simulink model "dss_ideal_16384ch_32bits_reset_1966mhz_cx"  (R2021a) (continued)
== system dss_ideal_16384ch_32bits_reset_1966mhz_cx == (continued)
      mask init:
      | shared_bram_mask;
      mask TypeOptions arith_type: Unsigned | Signed  (2's comp)
      mask TypeOptions data_width: 8 | 16 | 32 | 64 | 128
      mask TypeOptions optimization: Minimum_Area | Low_Power
  synth0_2 : Subsystem  Tag=xps:bram  AncestorBlock=xps_library/Memory/shared_bram  mask{arith_type=Unsigned, addr_width=11, data_width=32, reg_prim_output=on, reg_core_output=on, optimization=Minimum_Area, data_bin_pt=0, init_vals=[0:2^11-1], sample_rate=1}
      mask prompts: arith_type "Output Data Type"; addr_width "Address width"; data_width "Data Width"; reg_prim_output "Register Primitive Output"; reg_core_output "Register Core Output"; data_bin_pt "Data Binary Point"; init_vals "Initial values (simulation only)"; sample_rate "Sample rate"
      mask init:
      | shared_bram_mask;
      mask TypeOptions arith_type: Unsigned | Signed  (2's comp)
      mask TypeOptions data_width: 8 | 16 | 32 | 64 | 128
      mask TypeOptions optimization: Minimum_Area | Low_Power
  synth0_3 : Subsystem  Tag=xps:bram  AncestorBlock=xps_library/Memory/shared_bram  mask{arith_type=Unsigned, addr_width=11, data_width=32, reg_prim_output=on, reg_core_output=on, optimization=Minimum_Area, data_bin_pt=0, init_vals=[0:2^11-1], sample_rate=1}
      mask prompts: arith_type "Output Data Type"; addr_width "Address width"; data_width "Data Width"; reg_prim_output "Register Primitive Output"; reg_core_output "Register Core Output"; data_bin_pt "Data Binary Point"; init_vals "Initial values (simulation only)"; sample_rate "Sample rate"
      mask init:
      | shared_bram_mask;
      mask TypeOptions arith_type: Unsigned | Signed  (2's comp)
      mask TypeOptions data_width: 8 | 16 | 32 | 64 | 128
      mask TypeOptions optimization: Minimum_Area | Low_Power
  synth0_4 : Subsystem  Tag=xps:bram  AncestorBlock=xps_library/Memory/shared_bram  mask{arith_type=Unsigned, addr_width=11, data_width=32, reg_prim_output=on, reg_core_output=on, optimization=Minimum_Area, data_bin_pt=0, init_vals=[0:2^11-1], sample_rate=1}
      mask prompts: arith_type "Output Data Type"; addr_width "Address width"; data_width "Data Width"; reg_prim_output "Register Primitive Output"; reg_core_output "Register Core Output"; data_bin_pt "Data Binary Point"; init_vals "Initial values (simulation only)"; sample_rate "Sample rate"
      mask init:
      | shared_bram_mask;
      mask TypeOptions arith_type: Unsigned | Signed  (2's comp)
      mask TypeOptions data_width: 8 | 16 | 32 | 64 | 128
      mask TypeOptions optimization: Minimum_Area | Low_Power
  synth0_5 : Subsystem  Tag=xps:bram  AncestorBlock=xps_library/Memory/shared_bram  mask{arith_type=Unsigned, addr_width=11, data_width=32, reg_prim_output=on, reg_core_output=on, optimization=Minimum_Area, data_bin_pt=0, init_vals=[0:2^11-1], sample_rate=1}
      mask prompts: arith_type "Output Data Type"; addr_width "Address width"; data_width "Data Width"; reg_prim_output "Register Primitive Output"; reg_core_output "Register Core Output"; data_bin_pt "Data Binary Point"; init_vals "Initial values (simulation only)"; sample_rate "Sample rate"
      mask init:
      | shared_bram_mask;
      mask TypeOptions arith_type: Unsigned | Signed  (2's comp)
      mask TypeOptions data_width: 8 | 16 | 32 | 64 | 128
      mask TypeOptions optimization: Minimum_Area | Low_Power
  synth0_6 : Subsystem  Tag=xps:bram  AncestorBlock=xps_library/Memory/shared_bram  mask{arith_type=Unsigned, addr_width=11, data_width=32, reg_prim_output=on, reg_core_output=on, optimization=Minimum_Area, data_bin_pt=0, init_vals=[0:2^11-1], sample_rate=1}
      mask prompts: arith_type "Output Data Type"; addr_width "Address width"; data_width "Data Width"; reg_prim_output "Register Primitive Output"; reg_core_output "Register Core Output"; data_bin_pt "Data Binary Point"; init_vals "Initial values (simulation only)"; sample_rate "Sample rate"
      mask init:
      | shared_bram_mask;
      mask TypeOptions arith_type: Unsigned | Signed  (2's comp)
      mask TypeOptions data_width: 8 | 16 | 32 | 64 | 128
      mask TypeOptions optimization: Minimum_Area | Low_Power
  synth0_7 : Subsystem  Tag=xps:bram  AncestorBlock=xps_library/Memory/shared_bram  mask{arith_type=Unsigned, addr_width=11, data_width=32, reg_prim_output=on, reg_core_output=on, optimization=Minimum_Area, data_bin_pt=0, init_vals=[0:2^11-1], sample_rate=1}
      mask prompts: arith_type "Output Data Type"; addr_width "Address width"; data_width "Data Width"; reg_prim_output "Register Primitive Output"; reg_core_output "Register Core Output"; data_bin_pt "Data Binary Point"; init_vals "Initial values (simulation only)"; sample_rate "Sample rate"
      mask init:
      | shared_bram_mask;
      mask TypeOptions arith_type: Unsigned | Signed  (2's comp)
      mask TypeOptions data_width: 8 | 16 | 32 | 64 | 128
      mask TypeOptions optimization: Minimum_Area | Low_Power
  synth1_0 : Subsystem  Tag=xps:bram  AncestorBlock=xps_library/Memory/shared_bram  mask{arith_type=Unsigned, addr_width=11, data_width=32, reg_prim_output=on, reg_core_output=on, optimization=Minimum_Area, data_bin_pt=0, init_vals=[0:2^11-1], sample_rate=1}
      mask prompts: arith_type "Output Data Type"; addr_width "Address width"; data_width "Data Width"; reg_prim_output "Register Primitive Output"; reg_core_output "Register Core Output"; data_bin_pt "Data Binary Point"; init_vals "Initial values (simulation only)"; sample_rate "Sample rate"
      mask init:
      | shared_bram_mask;
      mask TypeOptions arith_type: Unsigned | Signed  (2's comp)
      mask TypeOptions data_width: 8 | 16 | 32 | 64 | 128
      mask TypeOptions optimization: Minimum_Area | Low_Power
  synth1_1 : Subsystem  Tag=xps:bram  AncestorBlock=xps_library/Memory/shared_bram  mask{arith_type=Unsigned, addr_width=11, data_width=32, reg_prim_output=on, reg_core_output=on, optimization=Minimum_Area, data_bin_pt=0, init_vals=[0:2^11-1], sample_rate=1}
      mask prompts: arith_type "Output Data Type"; addr_width "Address width"; data_width "Data Width"; reg_prim_output "Register Primitive Output"; reg_core_output "Register Core Output"; data_bin_pt "Data Binary Point"; init_vals "Initial values (simulation only)"; sample_rate "Sample rate"
      mask init:
      | shared_bram_mask;
      mask TypeOptions arith_type: Unsigned | Signed  (2's comp)
      mask TypeOptions data_width: 8 | 16 | 32 | 64 | 128
      mask TypeOptions optimization: Minimum_Area | Low_Power
  synth1_2 : Subsystem  Tag=xps:bram  AncestorBlock=xps_library/Memory/shared_bram  mask{arith_type=Unsigned, addr_width=11, data_width=32, reg_prim_output=on, reg_core_output=on, optimization=Minimum_Area, data_bin_pt=0, init_vals=[0:2^11-1], sample_rate=1}
      mask prompts: arith_type "Output Data Type"; addr_width "Address width"; data_width "Data Width"; reg_prim_output "Register Primitive Output"; reg_core_output "Register Core Output"; data_bin_pt "Data Binary Point"; init_vals "Initial values (simulation only)"; sample_rate "Sample rate"
      mask init:
      | shared_bram_mask;
      mask TypeOptions arith_type: Unsigned | Signed  (2's comp)
      mask TypeOptions data_width: 8 | 16 | 32 | 64 | 128
      mask TypeOptions optimization: Minimum_Area | Low_Power
  synth1_3 : Subsystem  Tag=xps:bram  AncestorBlock=xps_library/Memory/shared_bram  mask{arith_type=Unsigned, addr_width=11, data_width=32, reg_prim_output=on, reg_core_output=on, optimization=Minimum_Area, data_bin_pt=0, init_vals=[0:2^11-1], sample_rate=1}
      mask prompts: arith_type "Output Data Type"; addr_width "Address width"; data_width "Data Width"; reg_prim_output "Register Primitive Output"; reg_core_output "Register Core Output"; data_bin_pt "Data Binary Point"; init_vals "Initial values (simulation only)"; sample_rate "Sample rate"
      mask init:
      | shared_bram_mask;
      mask TypeOptions arith_type: Unsigned | Signed  (2's comp)
      mask TypeOptions data_width: 8 | 16 | 32 | 64 | 128
      mask TypeOptions optimization: Minimum_Area | Low_Power
  synth1_4 : Subsystem  Tag=xps:bram  AncestorBlock=xps_library/Memory/shared_bram  mask{arith_type=Unsigned, addr_width=11, data_width=32, reg_prim_output=on, reg_core_output=on, optimization=Minimum_Area, data_bin_pt=0, init_vals=[0:2^11-1], sample_rate=1}
      mask prompts: arith_type "Output Data Type"; addr_width "Address width"; data_width "Data Width"; reg_prim_output "Register Primitive Output"; reg_core_output "Register Core Output"; data_bin_pt "Data Binary Point"; init_vals "Initial values (simulation only)"; sample_rate "Sample rate"
      mask init:
      | shared_bram_mask;
      mask TypeOptions arith_type: Unsigned | Signed  (2's comp)
      mask TypeOptions data_width: 8 | 16 | 32 | 64 | 128
      mask TypeOptions optimization: Minimum_Area | Low_Power
  synth1_5 : Subsystem  Tag=xps:bram  AncestorBlock=xps_library/Memory/shared_bram  mask{arith_type=Unsigned, addr_width=11, data_width=32, reg_prim_output=on, reg_core_output=on, optimization=Minimum_Area, data_bin_pt=0, init_vals=[0:2^11-1], sample_rate=1}
      mask prompts: arith_type "Output Data Type"; addr_width "Address width"; data_width "Data Width"; reg_prim_output "Register Primitive Output"; reg_core_output "Register Core Output"; data_bin_pt "Data Binary Point"; init_vals "Initial values (simulation only)"; sample_rate "Sample rate"
      mask init:
      | shared_bram_mask;
      mask TypeOptions arith_type: Unsigned | Signed  (2's comp)
      mask TypeOptions data_width: 8 | 16 | 32 | 64 | 128
      mask TypeOptions optimization: Minimum_Area | Low_Power
  synth1_6 : Subsystem  Tag=xps:bram  AncestorBlock=xps_library/Memory/shared_bram  mask{arith_type=Unsigned, addr_width=11, data_width=32, reg_prim_output=on, reg_core_output=on, optimization=Minimum_Area, data_bin_pt=0, init_vals=[0:2^11-1], sample_rate=1}
      mask prompts: arith_type "Output Data Type"; addr_width "Address width"; data_width "Data Width"; reg_prim_output "Register Primitive Output"; reg_core_output "Register Core Output"; data_bin_pt "Data Binary Point"; init_vals "Initial values (simulation only)"; sample_rate "Sample rate"
      mask init:
      | shared_bram_mask;
      mask TypeOptions arith_type: Unsigned | Signed  (2's comp)
      mask TypeOptions data_width: 8 | 16 | 32 | 64 | 128
      mask TypeOptions optimization: Minimum_Area | Low_Power
  synth1_7 : Subsystem  Tag=xps:bram  AncestorBlock=xps_library/Memory/shared_bram  mask{arith_type=Unsigned, addr_width=11, data_width=32, reg_prim_output=on, reg_core_output=on, optimization=Minimum_Area, data_bin_pt=0, init_vals=[0:2^11-1], sample_rate=1}
      mask prompts: arith_type "Output Data Type"; addr_width "Address width"; data_width "Data Width"; reg_prim_output "Register Primitive Output"; reg_core_output "Register Core Output"; data_bin_pt "Data Binary Point"; init_vals "Initial values (simulation only)"; sample_rate "Sample rate"
      mask init:
      | shared_bram_mask;
      mask TypeOptions arith_type: Unsigned | Signed  (2's comp)
      mask TypeOptions data_width: 8 | 16 | 32 | 64 | 128
      mask TypeOptions optimization: Minimum_Area | Low_Power
  wires:
  rfdc/1(m00_axis_tdata) -> bus_create/1(in1)
  rfdc/2(m01_axis_tdata) -> bus_create/2(in2)
  bus_create/1(bus_out) -> munge1/1(din), bus_expand2/1(bus_in)
  bus_expand/8(lsb_out1) -> pfb_fir/9(pol1_in8)
  bus_expand/1(msb_out8) -> pfb_fir/2(pol1_in1)
  bus_expand/3(out6) -> pfb_fir/4(pol1_in3)
  pfb_fir/7(pol1_out6) -> fft/8(in05)
  pfb_fir/1(sync_out) -> fft/1(sync)
  pfb_fir/9(pol1_out8) -> fft/10(in07)
  bus_expand/4(out5) -> pfb_fir/5(pol1_in4)
  [sync_gen] -> pfb_fir/1(sync)
  pfb_fir/5(pol1_out4) -> fft/6(in03)
  Constant2/1 -> fft/2(shift)
  bus_expand/7(out2) -> pfb_fir/8(pol1_in7)
  bus_expand/5(out4) -> pfb_fir/6(pol1_in5)
  bus_expand/6(out3) -> pfb_fir/7(pol1_in6)
  pfb_fir/6(pol1_out5) -> fft/7(in04)
  bus_expand/2(out7) -> pfb_fir/3(pol1_in2)
  pfb_fir/4(pol1_out3) -> fft/5(in02)
  pfb_fir/2(pol1_out1) -> fft/3(in00)
  pfb_fir/8(pol1_out7) -> fft/9(in06)
  pfb_fir/3(pol1_out2) -> fft/4(in01)
  Band-Limited White Noise/1 -> rfdc/10(m23_axis_tdata_sim), rfdc/11(m30_axis_tdata_sim), rfdc/12(m32_axis_tdata_sim), rfdc/9(m22_axis_tdata_sim), rfdc/8(m21_axis_tdata_sim), rfdc/7(m20_axis_tdata_sim), rfdc/6(m12_axis_tdata_sim), rfdc/5(m10_axis_tdata_sim), rfdc/4(m03_axis_tdata_sim), rfdc/3(m02_axis_tdata_sim), rfdc/2(m01_axis_tdata_sim), rfdc/1(m00_axis_tdata_sim)
  rfdc/5(m10_axis_tdata) -> Terminator4/1
  rfdc/6(m12_axis_tdata) -> Terminator9/1
  rfdc/8(m21_axis_tdata) -> Terminator17/1
  rfdc/12(m32_axis_tdata) -> Terminator19/1
  rfdc/7(m20_axis_tdata) -> Terminator16/1
  rfdc/9(m22_axis_tdata) -> Terminator20/1
  rfdc/10(m23_axis_tdata) -> Terminator21/1
  rfdc/11(m30_axis_tdata) -> Terminator18/1
  pipeline157/1(q) -> comp_add/1(a)
  pipeline113/1(q) -> spect_cnt2/1
  simple_bram_vacc1/2(valid) -> spect_cnt2/2, pipeline118/1(d)
  pipeline161/1(q) -> Delay46/1
  pipeline126/1(q) -> simple_bram_vacc1/2(din)
  bram_mult0_0/1(c) -> comp_add/2(b)
  Delay46/1 -> Delay43/1
  Reinterpret21/1 -> pipeline117/1(d)
  simple_bram_vacc1/1(dout) -> Reinterpret21/1
  pipeline118/1(q) -> synth0_0/3(we)
  pipeline162/1(q) -> pipeline161/1(d)
  synth0_0/1(data_out) -> Terminator1/1
  pipeline125/1(q) -> simple_bram_vacc1/1(new_acc)
  comp_add/1(a+b) -> power1/1
  pipeline104/1(q) -> synth0_0/1(addr)
  [acc_len] -> acc_cntrl/1(acc_len)
  [sync_rst] -> acc_cntrl/3(rst)
  pipeline117/1(q) -> synth0_0/2(data_in)
  power1/1 -> Delay44/1
  fft/1(sync_out) -> Delay39/1
  Delay39/1 -> Delay38/1
  Delay38/1 -> pipeline162/1(d), bram_mult0_1/1(In1), bram_mult0_2/1(In1), bram_mult0_3/1(In1), bram_mult0_4/1(In1), bram_mult0_5/1(In1), bram_mult0_8/1(In1), bram_mult0_9/1(In1), bram_mult0_10/1(In1), bram_mult0_11/1(In1), bram_mult0_12/1(In1), bram_mult0_13/1(In1), bram_mult0_14/1(In1), bram_mult0_15/1(In1), bram_mult0_7/1(In1), bram_mult0_6/1(In1), bram_mult0_0/1(In1)
  Delay15/1 -> bram_mult0_8/2(d), pipeline157/1(d)
  fft/2(out00) -> Delay18/1
  Delay18/1 -> Delay15/1
  Delay3/1 -> bram_mult0_9/2(d), pipeline10/1(d)
  fft/3(out01) -> Delay4/1
  Delay4/1 -> Delay3/1
  Delay2/1 -> Delay1/1
  fft/4(out02) -> Delay2/1
  Delay1/1 -> bram_mult0_10/2(d), pipeline12/1(d)
  fft/6(out04) -> Delay6/1
  Delay8/1 -> Delay7/1
  Delay6/1 -> Delay5/1
  fft/5(out03) -> Delay8/1
  Delay5/1 -> bram_mult0_12/2(d), pipeline21/1(d)
  Delay7/1 -> bram_mult0_11/2(d), pipeline19/1(d)
  Delay10/1 -> Delay9/1
  Delay12/1 -> Delay11/1
  Delay13/1 -> bram_mult0_14/2(d), pipeline40/1(d)
  Delay11/1 -> bram_mult0_15/2(d), pipeline42/1(d)
  Delay9/1 -> bram_mult0_13/2(d), pipeline33/1(d)
  Delay14/1 -> Delay13/1
  pipeline1/1(q) -> synth0_1/1(addr)
  pipeline4/1(q) -> synth0_1/3(we)
  simple_bram_vacc2/1(dout) -> Reinterpret1/1
  pipeline2/1(q) -> synth0_1/2(data_in)
  pipeline6/1(q) -> simple_bram_vacc2/1(new_acc)
  bram_mult0_1/1(c) -> comp_add1/2(b)
  pipeline10/1(q) -> comp_add1/1(a)
  power2/1 -> Delay16/1
  synth0_1/1(data_out) -> Terminator3/1
  simple_bram_vacc2/2(valid) -> pipeline4/1(d)
  comp_add1/1(a+b) -> power2/1
  pipeline7/1(q) -> simple_bram_vacc2/2(din)
  Reinterpret1/1 -> pipeline2/1(d)
  Reinterpret2/1 -> pipeline13/1(d)
  pipeline12/1(q) -> comp_add2/1(a)
  simple_bram_vacc3/2(valid) -> pipeline14/1(d)
  power3/1 -> Delay17/1
  bram_mult0_2/1(c) -> comp_add2/2(b)
  synth0_2/1(data_out) -> Terminator5/1
  comp_add2/1(a+b) -> power3/1
  pipeline17/1(q) -> simple_bram_vacc3/2(din)
  pipeline13/1(q) -> synth0_2/2(data_in)
  simple_bram_vacc3/1(dout) -> Reinterpret2/1
  pipeline16/1(q) -> simple_bram_vacc3/1(new_acc)
  pipeline14/1(q) -> synth0_2/3(we)
  bram_mult0_4/1(c) -> comp_add4/2(b)
  pipeline27/1(q) -> synth0_3/2(data_in)
  comp_add4/1(a+b) -> power5/1
  pipeline31/1(q) -> simple_bram_vacc4/2(din)
  pipeline23/1(q) -> synth0_4/3(we)
  bram_mult0_3/1(c) -> comp_add3/2(b)
  Reinterpret3/1 -> pipeline27/1(d)
  pipeline19/1(q) -> comp_add3/1(a)
  power5/1 -> Delay20/1
  Reinterpret4/1 -> pipeline22/1(d)
  pipeline22/1(q) -> synth0_4/2(data_in)
  pipeline25/1(q) -> simple_bram_vacc5/1(new_acc)
  simple_bram_vacc4/2(valid) -> pipeline28/1(d)
  simple_bram_vacc5/2(valid) -> pipeline23/1(d)
  synth0_3/1(data_out) -> Terminator6/1
  synth0_4/1(data_out) -> Terminator7/1
  simple_bram_vacc4/1(dout) -> Reinterpret3/1
  pipeline30/1(q) -> simple_bram_vacc4/1(new_acc)
  power4/1 -> Delay19/1
  comp_add3/1(a+b) -> power4/1
  pipeline21/1(q) -> comp_add4/1(a)
  simple_bram_vacc5/1(dout) -> Reinterpret4/1
  pipeline26/1(q) -> simple_bram_vacc5/2(din)
  pipeline28/1(q) -> synth0_3/3(we)
  pipeline47/1(q) -> simple_bram_vacc8/2(din)
  pipeline51/1(q) -> simple_bram_vacc7/1(new_acc)
  simple_bram_vacc6/2(valid) -> pipeline35/1(d)
  pipeline33/1(q) -> comp_add5/1(a)
  simple_bram_vacc6/1(dout) -> Reinterpret5/1
  pipeline43/1(q) -> synth0_7/2(data_in)
  synth0_6/1(data_out) -> Terminator10/1
  power8/1 -> Delay24/1
  power7/1 -> Delay22/1
  simple_bram_vacc8/2(valid) -> pipeline44/1(d)
  simple_bram_vacc8/1(dout) -> Reinterpret7/1
  Reinterpret5/1 -> pipeline34/1(d)
  bram_mult0_5/1(c) -> comp_add5/2(b)
  comp_add6/1(a+b) -> power7/1
  pipeline48/1(q) -> synth0_6/2(data_in)
  simple_bram_vacc7/1(dout) -> Reinterpret6/1
  simple_bram_vacc7/2(valid) -> pipeline49/1(d)
  bram_mult0_7/1(c) -> comp_add7/2(b)
  pipeline44/1(q) -> synth0_7/3(we)
  pipeline34/1(q) -> synth0_5/2(data_in)
  synth0_7/1(data_out) -> Terminator11/1
  pipeline42/1(q) -> comp_add7/1(a)
  bram_mult0_6/1(c) -> comp_add6/2(b)
  pipeline52/1(q) -> simple_bram_vacc7/2(din)
  Reinterpret6/1 -> pipeline48/1(d)
  synth0_5/1(data_out) -> Terminator8/1
  pipeline37/1(q) -> simple_bram_vacc6/1(new_acc)
  pipeline46/1(q) -> simple_bram_vacc8/1(new_acc)
  Reinterpret7/1 -> pipeline43/1(d)
  pipeline35/1(q) -> synth0_5/3(we)
  comp_add5/1(a+b) -> power6/1
  pipeline49/1(q) -> synth0_6/3(we)
  comp_add7/1(a+b) -> power8/1
  pipeline38/1(q) -> simple_bram_vacc6/2(din)
  pipeline40/1(q) -> comp_add6/1(a)
  power6/1 -> Delay21/1
  Delay57/1 -> Delay56/1
  simple_bram_vacc13/1(dout) -> Reinterpret12/1
  pipeline76/1(q) -> comp_add12/1(a)
  fft/15(out15) -> Delay26/1
  Delay55/1 -> Delay54/1
  pipeline99/1(q) -> comp_add15/1(a)
  bus_expand1/2(out7) -> pfb_fir/11(pol2_in2)
  synth1_5/1(data_out) -> Terminator25/1
  bram_mult0_14/1(c) -> comp_add14/2(b)
  power13/1 -> Delay37/1
  simple_bram_vacc16/1(dout) -> Reinterpret15/1
  fft/16(out16) -> Delay30/1
  pipeline94/1(q) -> simple_bram_vacc14/2(din)
  fft/11(out11) -> Delay50/1
  Delay35/1 -> Delay220/1
  pipeline55/1(q) -> synth1_0/1(addr)
  pipeline80/1(q) -> simple_bram_vacc13/1(new_acc)
  pipeline89/1(q) -> comp_add13/1(a)
  Delay58/1 -> bram_mult0_5/2(d), pipeline89/1(d)
  pipeline86/1(q) -> simple_bram_vacc12/1(new_acc)
  Delay54/1 -> bram_mult0_4/2(d), pipeline76/1(d)
  pipeline97/1(q) -> comp_add14/1(a)
  simple_bram_vacc9/1(dout) -> Reinterpret10/1
  simple_bram_vacc10/1(dout) -> Reinterpret8/1
  pfb_fir/11(pol2_out2) -> fft/12(in11)
  Delay34/1 -> Delay31/1
  power14/1 -> Delay40/1
  simple_bram_vacc14/2(valid) -> pipeline91/1(d)
  simple_bram_vacc15/1(dout) -> Reinterpret14/1
  simple_bram_vacc9/2(valid) -> pipeline59/1(d)
  Delay41/1 -> Delay222/1
  simple_bram_vacc12/2(valid) -> pipeline83/1(d)
  power15/1 -> Delay41/1
  Reinterpret13/1 -> pipeline90/1(d)
  Delay33/1 -> Delay219/1
  fft/10(out10) -> Delay34/1
  bus_expand1/8(lsb_out1) -> pfb_fir/17(pol2_in8)
  simple_bram_vacc13/2(valid) -> pipeline78/1(d)
  pipeline62/1(q) -> simple_bram_vacc9/1(new_acc)
  bram_mult0_11/1(c) -> comp_add11/2(b)
  Reinterpret9/1 -> pipeline64/1(d)
  Delay37/1 -> Delay221/1
  pipeline105/1(q) -> simple_bram_vacc16/2(din)
  pipeline87/1(q) -> simple_bram_vacc12/2(din)
  simple_bram_vacc16/2(valid) -> pipeline101/1(d)
  pfb_fir/12(pol2_out3) -> fft/13(in12)
  pipeline68/1(q) -> simple_bram_vacc11/1(new_acc)
  power12/1 -> Delay35/1
  pfb_fir/17(pol2_out8) -> fft/18(in17)
  fft/12(out12) -> Delay36/1
  pipeline65/1(q) -> synth1_2/3(we)
  pipeline91/1(q) -> synth1_5/3(we)
  Reinterpret8/1 -> pipeline74/1(d)
  bram_mult0_12/1(c) -> comp_add12/2(b)
  bus_expand1/3(out6) -> pfb_fir/12(pol2_in3)
  fft/14(out14) -> Delay55/1
  pipeline90/1(q) -> synth1_5/2(data_in)
  pipeline107/1(q) -> synth1_6/3(we)
  fft/17(out17) -> Delay28/1
  synth1_1/1(data_out) -> Terminator15/1
  pfb_fir/14(pol2_out5) -> fft/15(in14)
  bram_mult0_10/1(c) -> comp_add10/2(b)
  pipeline106/1(q) -> synth1_6/2(data_in)
  pipeline63/1(q) -> simple_bram_vacc9/2(din)
  Reinterpret14/1 -> pipeline106/1(d)
  pipeline71/1(q) -> simple_bram_vacc11/2(din)
  simple_bram_vacc11/2(valid) -> pipeline65/1(d)
  Delay56/1 -> bram_mult0_3/2(d), pipeline73/1(d)
  pipeline59/1(q) -> synth1_0/3(we)
  pipeline78/1(q) -> synth1_4/3(we)
  bram_mult0_8/1(c) -> comp_add8/2(b)
  simple_bram_vacc15/2(valid) -> pipeline107/1(d)
  pfb_fir/13(pol2_out4) -> fft/14(in13)
  bram_mult0_13/1(c) -> comp_add13/2(b)
  pipeline77/1(q) -> synth1_4/2(data_in)
  bus_expand1/5(out4) -> pfb_fir/14(pol2_in5)
  bus_expand1/1(msb_out8) -> pfb_fir/10(pol2_in1)
  Delay29/1 -> bram_mult0_6/2(d), pipeline97/1(d)
  Delay27/1 -> pipeline99/1(d), bram_mult0_7/2(d)
  Delay50/1 -> Delay47/1
  Reinterpret11/1 -> pipeline82/1(d)
  synth1_7/1(data_out) -> Terminator14/1
  Delay26/1 -> Delay58/1
  Reinterpret15/1 -> pipeline100/1(d)
  pipeline82/1(q) -> synth1_3/2(data_in)
  power9/1 -> Delay52/1
  simple_bram_vacc14/1(dout) -> Reinterpret13/1
  pipeline54/1(q) -> comp_add9/1(a)
  Reinterpret10/1 -> pipeline58/1(d)
  pipeline64/1(q) -> synth1_2/2(data_in)
  power10/1 -> Delay32/1
  Delay47/1 -> bram_mult0_1/2(d), pipeline54/1(d)
  pipeline101/1(q) -> synth1_7/3(we)
  bram_mult0_15/1(c) -> comp_add15/2(b)
  simple_bram_vacc12/1(dout) -> Reinterpret11/1
  power11/1 -> Delay33/1
  fft/13(out13) -> Delay57/1
  Delay36/1 -> Delay25/1
  Reinterpret12/1 -> pipeline77/1(d)
  synth1_2/1(data_out) -> Terminator22/1
  pipeline93/1(q) -> simple_bram_vacc14/1(new_acc)
  Delay30/1 -> Delay29/1
  simple_bram_vacc10/2(valid) -> pipeline96/1(d)
  Delay45/1 -> Delay225/1
  pipeline67/1(q) -> comp_add8/1(a)
  Delay40/1 -> Delay226/1
  bus_expand1/7(out2) -> pfb_fir/16(pol2_in7)
  synth1_6/1(data_out) -> Terminator13/1
  pipeline58/1(q) -> synth1_0/2(data_in)
  pipeline100/1(q) -> synth1_7/2(data_in)
  synth1_3/1(data_out) -> Terminator23/1
  pipeline53/1(q) -> synth1_1/1(addr)
  pipeline60/1(q) -> comp_add10/1(a)
  power16/1 -> Delay45/1
  pipeline114/1(q) -> simple_bram_vacc10/2(din)
  bus_expand1/6(out3) -> pfb_fir/15(pol2_in6)
  Delay31/1 -> bram_mult0_0/2(d), pipeline67/1(d)
  pipeline81/1(q) -> simple_bram_vacc13/2(din)
  bram_mult0_9/1(c) -> comp_add9/2(b)
  simple_bram_vacc11/1(dout) -> Reinterpret9/1
  pipeline83/1(q) -> synth1_3/3(we)
  pipeline112/1(q) -> simple_bram_vacc10/1(new_acc)
  synth1_4/1(data_out) -> Terminator24/1
  Delay28/1 -> Delay27/1
  pipeline103/1(q) -> simple_bram_vacc16/1(new_acc)
  Delay25/1 -> bram_mult0_2/2(d), pipeline60/1(d)
  pfb_fir/10(pol2_out1) -> fft/11(in10)
  pipeline74/1(q) -> synth1_1/2(data_in)
  pipeline73/1(q) -> comp_add11/1(a)
  pfb_fir/16(pol2_out7) -> fft/17(in16)
  pipeline111/1(q) -> simple_bram_vacc15/2(din)
  pfb_fir/15(pol2_out6) -> fft/16(in15)
  pipeline110/1(q) -> simple_bram_vacc15/1(new_acc)
  pipeline96/1(q) -> synth1_1/3(we)
  bus_expand1/4(out5) -> pfb_fir/13(pol2_in4)
  synth1_0/1(data_out) -> Terminator12/1
  rfdc/3(m02_axis_tdata) -> bus_create1/1(in1)
  rfdc/4(m03_axis_tdata) -> bus_create1/2(in2)
  pipeline11/1(q) -> synth0_2/1(addr)
  pipeline18/1(q) -> synth0_3/1(addr)
  pipeline20/1(q) -> synth0_4/1(addr)
  pipeline32/1(q) -> synth0_5/1(addr)
  pipeline39/1(q) -> synth0_6/1(addr)
  pipeline41/1(q) -> synth0_7/1(addr)
  pipeline56/1(q) -> synth1_2/1(addr)
  pipeline72/1(q) -> synth1_3/1(addr)
  pipeline75/1(q) -> synth1_4/1(addr)
  pipeline88/1(q) -> synth1_5/1(addr)
  pipeline95/1(q) -> synth1_6/1(addr)
  pipeline98/1(q) -> synth1_7/1(addr)
  adc1_snapshot0/1(arm_out) -> Terminator28/1
  Reinterpret17/1 -> adc1_snapshot0/1(in_m00)
  bus_expand2/2(lsb_out1) -> Reinterpret16/1
  adc1_snapshot1/1(arm_out) -> Terminator29/1
  bus_expand2/1(msb_out2) -> Reinterpret17/1
  Constant4/1 -> adc1_snapshot1/3(trig), adc1_snapshot1/2(we)
  Constant3/1 -> adc1_snapshot0/3(trig), adc1_snapshot0/2(we)
  Reinterpret18/1 -> adc1_snapshot3/1(in_m03)
  bus_expand3/2(lsb_out1) -> Reinterpret18/1
  adc1_snapshot2/1(arm_out) -> Terminator30/1
  bus_create1/1(bus_out) -> bus_expand3/1(bus_in), munge2/1(din)
  bus_expand3/1(msb_out2) -> Reinterpret19/1
  Reinterpret19/1 -> adc1_snapshot2/1(in_m02)
  Constant5/1 -> adc1_snapshot2/3(trig), adc1_snapshot2/2(we)
  Constant6/1 -> adc1_snapshot3/3(trig), adc1_snapshot3/2(we)
  adc1_snapshot3/1(arm_out) -> Terminator31/1
  comp_add9/1(a+b) -> power10/1
  comp_add8/1(a+b) -> power9/1
  comp_add10/1(a+b) -> power11/1
  comp_add11/1(a+b) -> power12/1
  comp_add12/1(a+b) -> power13/1
  comp_add13/1(a+b) -> power14/1
  comp_add14/1(a+b) -> power15/1
  comp_add15/1(a+b) -> power16/1
  Constant16/1 -> acc_len/1(sim_1)
  pipeline435/1(q) -> [cnt_rst]
  Constant19/1 -> cnt_rst/1(sim_1)
  cnt_rst/1(in_reg) -> pipeline435/1(d)
  acc_len/1(in_reg) -> pipeline9/1(d)
  pipeline9/1(q) -> [acc_len]
  pipeline121/1(q) -> pipeline126/1(d)
  pipeline5/1(q) -> pipeline7/1(d)
  pipeline15/1(q) -> pipeline17/1(d)
  pipeline29/1(q) -> pipeline31/1(d)
  pipeline24/1(q) -> pipeline26/1(d)
  pipeline45/1(q) -> pipeline47/1(d)
  pipeline36/1(q) -> pipeline38/1(d)
  pipeline50/1(q) -> pipeline52/1(d)
  pipeline108/1(q) -> pipeline114/1(d)
  pipeline102/1(q) -> pipeline105/1(d)
  pipeline109/1(q) -> pipeline111/1(d)
  pipeline79/1(q) -> pipeline81/1(d)
  pipeline92/1(q) -> pipeline94/1(d)
  pipeline66/1(q) -> pipeline71/1(d)
  pipeline84/1(q) -> pipeline87/1(d)
  pipeline61/1(q) -> pipeline63/1(d)
  Delay17/1 -> Delay155/1
  Delay154/1 -> round0/1(din)
  Delay53/1 -> round1/1(din)
  Delay20/1 -> Delay164/1
  Delay51/1 -> Delay223/1
  Delay19/1 -> Delay162/1
  Delay44/1 -> Delay154/1
  Delay24/1 -> Delay216/1
  Delay16/1 -> Delay53/1
  Delay155/1 -> round2/1(din)
  Delay224/1 -> Delay51/1
  Delay162/1 -> round3/1(din)
  Delay216/1 -> round7/1(din)
  Delay164/1 -> round4/1(din)
  Delay217/1 -> round5/1(din)
  [quant_gain] -> round0/2(scale), round1/2(scale), round2/2(scale), round3/2(scale), round4/2(scale), round5/2(scale), round6/2(scale), round7/2(scale), round8/2(scale), round9/2(scale), round10/2(scale), round11/2(scale), round12/2(scale), round13/2(scale), round14/2(scale), round15/2(scale)
  Delay223/1 -> acc_cntrl/2(sync)
  Delay21/1 -> Delay217/1
  Delay43/1 -> Delay224/1
  Delay22/1 -> Delay215/1
  Delay215/1 -> round6/1(din)
  round7/1(dout) -> pipeline45/1(d)
  round6/1(dout) -> pipeline50/1(d)
  round5/1(dout) -> pipeline36/1(d)
  round4/1(dout) -> pipeline24/1(d)
  round3/1(dout) -> pipeline29/1(d)
  round2/1(dout) -> pipeline15/1(d)
  round1/1(dout) -> pipeline5/1(d)
  round0/1(dout) -> pipeline121/1(d)
  gain/1(in_reg) -> pipeline57/1(d)
  Constant45/1 -> gain/1(sim_1)
  pipeline57/1(q) -> [quant_gain]
  round8/1(dout) -> pipeline61/1(d)
  Delay52/1 -> Delay218/1
  Delay32/1 -> Delay227/1
  round9/1(dout) -> pipeline108/1(d)
  round10/1(dout) -> pipeline66/1(d)
  round11/1(dout) -> pipeline84/1(d)
  round12/1(dout) -> pipeline79/1(d)
  round13/1(dout) -> pipeline92/1(d)
  round14/1(dout) -> pipeline109/1(d)
  round15/1(dout) -> pipeline102/1(d)
  Delay221/1 -> round12/1(din)
  Delay220/1 -> round11/1(din)
  Delay226/1 -> round13/1(din)
  Delay222/1 -> round14/1(din)
  Delay227/1 -> round9/1(din)
  Delay218/1 -> round8/1(din)
  Delay225/1 -> round15/1(din)
  Delay219/1 -> round10/1(din)
  Delay23/1 -> pipeline3/1(d)
  pipeline3/1(q) -> [sync_gen]
  sync_gen/1(sync_out) -> Delay23/1
  edge_detect/1(out) -> [sync_rst]
  Constant10/1 -> gpio/1
  gpio/1 -> edge_detect/1(in)
  [sync_rst] -> sync_cntr/2
  rst_cnt/1(sim_out_reg) -> Terminator85/1
  sync_cntr/1 -> Delay107/1
  Delay107/1 -> rst_cnt/1(out_reg)
  pipeline/1(q) -> pulse_ext1/1(in)
  [cnt_rst] -> sync_cntr/1
  pulse_ext1/1(out) -> pipeline219/1(d)
  [sync_rst] -> pipeline/1(d)
  led0_sync/1 -> Terminator84/1
  pipeline219/1(q) -> led0_sync/1
  [sync_rst] -> acc_cycle/1
  acc_cnt/1(sim_out_reg) -> Terminator86/1
  acc_cntr/1 -> Delay133/1
  Delay213/1 -> Relational1/1, Register2/1, acc_per_cycle/1(out_reg)
  Constant13/1 -> Relational4/1
  Register2/1 -> Relational4/2
  Relational1/1 -> Logical/2
  [cnt_rst] -> acc_cntr/1
  Logical/1 -> acc_cntr/2
  acc_per_cycle/1(sim_out_reg) -> Terminator81/1
  acc_cycle/1 -> Delay213/1
  Delay214/1 -> acc_cycle/2
  Delay133/1 -> acc_cnt/1(out_reg)
  Relational4/1 -> Logical/1
  Constant/1 -> Relational1/2
  Reinterpret16/1 -> adc1_snapshot1/1(in_m01)
  acc_cntrl/1(new_acc) -> Delay214/1, pipeline113/1(d), pipeline110/1(d), pipeline103/1(d), pipeline93/1(d), pipeline80/1(d), pipeline86/1(d), pipeline68/1(d), pipeline112/1(d), pipeline62/1(d), pipeline46/1(d), pipeline51/1(d), pipeline37/1(d), pipeline25/1(d), pipeline30/1(d), pipeline16/1(d), pipeline6/1(d), pipeline125/1(d)
  spect_cnt2/1 -> pipeline104/1(d), pipeline53/1(d), pipeline56/1(d), pipeline72/1(d), pipeline75/1(d), pipeline88/1(d), pipeline95/1(d), pipeline98/1(d), pipeline55/1(d), pipeline41/1(d), pipeline39/1(d), pipeline32/1(d), pipeline20/1(d), pipeline18/1(d), pipeline11/1(d), pipeline1/1(d)
  fft/7(out05) -> Delay10/1
  fft/8(out06) -> Delay14/1
  fft/9(out07) -> Delay12/1
  fft/18(of) -> Terminator2/1
  Delay117/1 -> bram_mult0_17/2(d), pipeline191/1(d)
  Delay87/1 -> Delay120/1
  Delay95/1 -> bram_mult0_29/2(d), pipeline235/1(d)
  round21/1(dout) -> pipeline228/1(d)
  Delay119/1 -> Delay117/1
  Reinterpret29/1 -> pipeline134/1(d)
  bus_expand5/5(out4) -> pfb_fir1/14(pol2_in5)
  pipeline223/1(q) -> simple_bram_vacc28/2(din)
  Delay99/1 -> Delay97/1
  pipeline146/1(q) -> pipeline145/1(d)
  acc_cntr1/1 -> Delay61/1
  pipeline172/1(q) -> simple_bram_vacc22/1(new_acc)
  pfb_fir1/6(pol1_out5) -> fft1/7(in04)
  bram_mult0_16/1(c) -> comp_add16/2(b)
  simple_bram_vacc29/2(valid) -> pipeline214/1(d)
  comp_add24/1(a+b) -> power26/1
  simple_bram_vacc31/2(valid) -> pipeline123/1(d)
  fft1/17(out17) -> Delay96/1
  comp_add25/1(a+b) -> power27/1
  Delay48/1 -> Delay131/1
  simple_bram_vacc23/2(valid) -> pipeline185/1(d)
  pfb_fir1/14(pol2_out5) -> fft1/15(in14)
  pipeline116/1(q) -> simple_bram_vacc32/1(new_acc)
  fft1/18(of) -> Terminator26/1
  bus_expand4/6(out3) -> pfb_fir1/7(pol1_in6)
  simple_bram_vacc25/2(valid) -> pipeline195/1(d)
  pfb_fir1/5(pol1_out4) -> fft1/6(in03)
  pipeline214/1(q) -> re_bin_synth1_4/3(we)
  Delay65/1 -> round24/1(din)
  pfb_fir1/1(sync_out) -> fft1/1(sync)
  Delay72/1 -> Delay42/1
  pipeline229/1(q) -> simple_bram_vacc30/1(new_acc)
  pipeline144/1(q) -> simple_bram_vacc19/1(new_acc)
  pfb_fir1/8(pol1_out7) -> fft1/9(in06)
  fft1/5(out03) -> Delay130/1
  pipeline227/1(q) -> re_bin_synth1_5/3(we)
  pipeline122/1(q) -> re_bin_synth1_6/2(data_in)
  Delay77/1 -> round28/1(din)
  bram_mult0_29/1(c) -> comp_add29/2(b)
  pipeline200/1(q) -> simple_bram_vacc25/2(din)
  Delay81/1 -> round18/1(din)
  simple_bram_vacc32/1(dout) -> Reinterpret27/1
  pipeline124/1(q) -> pipeline133/1(d)
  fft1/4(out02) -> Delay72/1
  simple_bram_vacc20/1(dout) -> Reinterpret30/1
  Reinterpret22/1 -> pipeline194/1(d)
  round26/1(dout) -> pipeline155/1(d)
  pipeline139/1(q) -> simple_bram_vacc17/2(din)
  simple_bram_vacc19/2(valid) -> pipeline141/1(d)
  bus_expand5/7(out2) -> pfb_fir1/16(pol2_in7)
  pipeline221/1(q) -> pipeline223/1(d)
  Logical1/1 -> acc_cntr1/2
  Delay121/1 -> Delay80/1
  pfb_fir1/10(pol2_out1) -> fft1/11(in10)
  Delay80/1 -> round30/1(din)
  pipeline204/1(q) -> comp_add30/1(a)
  Delay83/1 -> round19/1(din)
  simple_bram_vacc23/1(dout) -> Reinterpret33/1
  Delay125/1 -> bram_mult0_25/2(d), pipeline209/1(d)
  pipeline182/1(q) -> simple_bram_vacc24/1(new_acc)
  simple_bram_vacc24/1(dout) -> Reinterpret34/1
  pipeline231/1(q) -> re_bin_synth1_6/1(addr)
  Delay90/1 -> round31/1(din)
  simple_bram_vacc20/2(valid) -> pipeline160/1(d)
  simple_bram_vacc18/2(valid) -> pipeline175/1(d)
  Reinterpret35/1 -> pipeline210/1(d)
  bram_mult0_19/1(c) -> comp_add19/2(b)
  Delay106/1 -> Delay84/1
  pfb_fir1/13(pol2_out4) -> fft1/14(in13)
  pipeline177/1(q) -> re_bin_synth0_7/1(addr)
  fft1/1(sync_out) -> Delay109/1
  Delay98/1 -> bram_mult0_31/2(d), pipeline69/1(d)
  power23/1 -> Delay112/1
  pipeline147/1(q) -> simple_bram_vacc19/2(din)
  pipeline8/1(q) -> re_bin_synth0_1/1(addr)
  fft1/9(out07) -> Delay59/1
  Delay112/1 -> Delay85/1
  pipeline184/1(q) -> re_bin_synth0_6/2(data_in)
  Delay79/1 -> round27/1(din)
  Delay120/1 -> Delay86/1
  pipeline233/1(q) -> comp_add22/1(a)
  fft1/15(out15) -> Delay94/1
  Delay122/1 -> round17/1(din)
  pfb_fir1/4(pol1_out3) -> fft1/5(in02)
  pipeline167/1(q) -> re_bin_synth0_5/1(addr)
  power29/1 -> Delay74/1
  simple_bram_vacc17/1(dout) -> Reinterpret29/1
  simple_bram_vacc19/1(dout) -> Reinterpret28/1
  pipeline201/1(q) -> re_bin_synth1_2/2(data_in)
  Delay113/1 -> Delay87/1
  acc_cycle1/1 -> Delay75/1
  pipeline209/1(q) -> comp_add19/1(a)
  re_bin_synth1_6/1(data_out) -> Terminator35/1
  pipeline134/1(q) -> re_bin_synth0_0/2(data_in)
  pipeline131/1(q) -> simple_bram_vacc26/1(new_acc)
  simple_bram_vacc28/1(dout) -> Reinterpret23/1
  power22/1 -> Delay111/1
  Delay126/1 -> Delay125/1
  re_bin_synth0_1/1(data_out) -> Terminator42/1
  pipeline192/1(q) -> re_bin_synth1_0/1(addr)
  comp_add19/1(a+b) -> power20/1
  pfb_fir1/3(pol1_out2) -> fft1/4(in01)
  fft1/13(out13) -> Delay126/1
  Constant7/1 -> Relational3/1
  Delay100/1 -> bram_mult0_16/2(d), pipeline204/1(d)
  pipeline156/1(q) -> simple_bram_vacc21/1(new_acc)
  Delay130/1 -> Delay129/1
  simple_bram_vacc26/2(valid) -> pipeline232/1(d)
  pipeline143/1(q) -> comp_add16/1(a)
  pipeline228/1(q) -> pipeline230/1(d)
  pipeline123/1(q) -> re_bin_synth1_6/3(we)
  re_bin_synth1_7/1(data_out) -> Terminator36/1
  round28/1(dout) -> pipeline187/1(d)
  pipeline208/1(q) -> re_bin_synth1_3/1(addr)
  pipeline148/1(q) -> re_bin_synth0_3/1(addr)
  simple_bram_vacc21/2(valid) -> pipeline154/1(d)
  power25/1 -> Delay66/1
  Relational3/1 -> Logical1/1
  pipeline185/1(q) -> re_bin_synth0_6/3(we)
  simple_bram_vacc22/1(dout) -> Reinterpret32/1
  power21/1 -> Delay106/1
  pipeline217/1(q) -> simple_bram_vacc29/2(din)
  simple_bram_vacc27/1(dout) -> Reinterpret36/1
  Delay93/1 -> bram_mult0_24/2(d), pipeline197/1(d)
  Delay97/1 -> bram_mult0_28/2(d), pipeline233/1(d)
  Reinterpret24/1 -> pipeline213/1(d)
  pipeline189/1(q) -> simple_bram_vacc23/2(din)
  pipeline225/1(q) -> comp_add21/1(a)
  bram_mult0_22/1(c) -> comp_add22/2(b)
  simple_bram_vacc25/1(dout) -> Reinterpret22/1
  Delay59/1 -> Delay49/1
  fft1/11(out11) -> Delay119/1
  pipeline191/1(q) -> comp_add31/1(a)
  pipeline194/1(q) -> re_bin_synth1_0/2(data_in)
  pfb_fir1/7(pol1_out6) -> fft1/8(in05)
  bram_mult0_31/1(c) -> comp_add31/2(b)
  Delay89/1 -> round21/1(din)
  simple_bram_vacc22/2(valid) -> pipeline170/1(d)
  bram_mult0_30/1(c) -> comp_add30/2(b)
  round18/1(dout) -> pipeline203/1(d)
  Delay111/1 -> Delay89/1
  round20/1(dout) -> pipeline215/1(d)
  re_bin_synth0_7/1(data_out) -> Terminator33/1
  power32/1 -> Delay121/1
  pipeline132/1(q) -> spect_cnt1/1
  bus_expand4/8(lsb_out1) -> pfb_fir1/9(pol1_in8)
  Delay63/1 -> bram_mult0_30/2(d), pipeline143/1(d)
  Delay84/1 -> round20/1(din)
  pipeline178/1(q) -> comp_add29/1(a)
  pipeline168/1(q) -> comp_add27/1(a)
  comp_add30/1(a+b) -> power32/1
  pfb_fir1/16(pol2_out7) -> fft1/17(in16)
  Delay118/1 -> bram_mult0_20/2(d), pipeline152/1(d)
  re_bin_synth1_0/1(data_out) -> Terminator34/1
  Delay73/1 -> Delay68/1
  re_bin_synth0_6/1(data_out) -> Terminator32/1
  pipeline218/1(q) -> re_bin_synth1_3/2(data_in)
  Delay96/1 -> Delay95/1
  pfb_fir1/2(pol1_out1) -> fft1/3(in00)
  Reinterpret34/1 -> pipeline179/1(d)
  pipeline163/1(q) -> pipeline166/1(d)
  pipeline203/1(q) -> pipeline207/1(d)
  power31/1 -> Delay92/1
  Delay104/1 -> Delay83/1
  Delay75/1 -> Relational2/1, Register1/1, acc_per_cycle1/1(out_reg)
  bus_expand5/1(msb_out8) -> pfb_fir1/10(pol2_in1)
  bram_mult0_28/1(c) -> comp_add28/2(b)
  pipeline141/1(q) -> re_bin_synth0_2/3(we)
  pipeline170/1(q) -> re_bin_synth0_5/3(we)
  pipeline193/1(q) -> re_bin_synth1_2/1(addr)
  Reinterpret27/1 -> pipeline70/1(d)
  Delay62/1 -> Delay60/1
  pipeline165/1(q) -> simple_bram_vacc20/1(new_acc)
  pipeline234/1(q) -> re_bin_synth1_7/1(addr)
  comp_add17/1(a+b) -> power25/1
  [sync_rst] -> acc_cntrl1/3(rst)
  pipeline129/1(q) -> simple_bram_vacc31/1(new_acc)
  pipeline154/1(q) -> re_bin_synth0_4/3(we)
  bram_mult0_18/1(c) -> comp_add18/2(b)
  Delay61/1 -> acc_cnt1/1(out_reg)
  pipeline166/1(q) -> simple_bram_vacc20/2(din)
  pipeline151/1(q) -> re_bin_synth0_4/1(addr)
  pipeline119/1(q) -> re_bin_synth0_0/1(addr)
  pipeline195/1(q) -> re_bin_synth1_0/3(we)
  simple_bram_vacc30/1(dout) -> Reinterpret25/1
  pipeline207/1(q) -> simple_bram_vacc27/2(din)
  pipeline158/1(q) -> simple_bram_vacc21/2(din)
  Reinterpret26/1 -> pipeline122/1(d)
  pfb_fir1/9(pol1_out8) -> fft1/10(in07)
  bus_expand4/5(out4) -> pfb_fir1/6(pol1_in5)
  bram_mult0_27/1(c) -> comp_add27/2(b)
  Delay85/1 -> round22/1(din)
  pipeline174/1(q) -> re_bin_synth0_6/1(addr)
  pipeline202/1(q) -> re_bin_synth1_2/3(we)
  re_bin_synth0_4/1(data_out) -> Terminator45/1
  pipeline169/1(q) -> re_bin_synth0_5/2(data_in)
  pipeline220/1(q) -> re_bin_synth1_3/3(we)
  pipeline149/1(q) -> comp_add25/1(a)
  round31/1(dout) -> pipeline124/1(d)
  Delay102/1 -> Delay81/1
  re_bin_synth0_0/1(data_out) -> Terminator27/1
  round30/1(dout) -> pipeline198/1(d)
  Delay131/1 -> bram_mult0_21/2(d), pipeline168/1(d)
  power26/1 -> Delay69/1
  pipeline183/1(q) -> simple_bram_vacc24/2(din)
  simple_bram_vacc31/1(dout) -> Reinterpret26/1
  fft1/6(out04) -> Delay128/1
  power27/1 -> Delay71/1
  simple_bram_vacc17/2(valid) -> spect_cnt1/2, pipeline135/1(d)
  bram_mult0_20/1(c) -> comp_add20/2(b)
  bus_expand5/8(lsb_out1) -> pfb_fir1/17(pol2_in8)
  bus_expand4/4(out5) -> pfb_fir1/5(pol1_in4)
  [acc_len1] -> acc_cntrl1/1(acc_len)
  pipeline115/1(q) -> pipeline120/1(d)
  fft1/3(out01) -> Delay110/1
  re_bin_synth0_5/1(data_out) -> Terminator46/1
  pipeline213/1(q) -> re_bin_synth1_4/2(data_in)
  Relational2/1 -> Logical1/2
  [sync_rst] -> acc_cycle1/1
  round16/1(dout) -> pipeline137/1(d)
  Delay64/1 -> round16/1(din)
  munge2/1(dout) -> bus_expand1/1(bus_in), bus_expand5/1(bus_in)
  pipeline179/1(q) -> re_bin_synth0_7/2(data_in)
  pipeline181/1(q) -> pipeline183/1(d)
  round22/1(dout) -> pipeline127/1(d)
  bus_expand4/2(out7) -> pfb_fir1/3(pol1_in2)
  Delay116/1 -> Delay113/1
  Delay114/1 -> Delay64/1
  round24/1(dout) -> pipeline142/1(d)
  pipeline224/1(q) -> re_bin_synth1_5/1(addr)
  power18/1 -> Delay101/1
  simple_bram_vacc21/1(dout) -> Reinterpret31/1
  pipeline212/1(q) -> comp_add20/1(a)
  pipeline140/1(q) -> re_bin_synth0_2/2(data_in)
  Reinterpret30/1 -> pipeline159/1(d)
  Reinterpret20/1 -> pipeline150/1(d)
  pipeline175/1(q) -> re_bin_synth0_1/3(we)
  pipeline70/1(q) -> re_bin_synth1_7/2(data_in)
  round25/1(dout) -> pipeline163/1(d)
  acc_per_cycle1/1(sim_out_reg) -> Terminator47/1
  pipeline120/1(q) -> simple_bram_vacc32/2(din)
  pipeline198/1(q) -> pipeline200/1(d)
  pipeline199/1(q) -> simple_bram_vacc25/1(new_acc)
  bram_mult0_21/1(c) -> comp_add21/2(b)
  re_bin_synth1_3/1(data_out) -> Terminator39/1
  Delay82/1 -> Delay77/1
  pipeline133/1(q) -> simple_bram_vacc26/2(din)
  Delay115/1 -> Delay88/1
  pipeline190/1(q) -> re_bin_synth1_1/1(addr)
  pipeline160/1(q) -> re_bin_synth0_3/3(we)
  pipeline152/1(q) -> comp_add26/1(a)
  simple_bram_vacc27/2(valid) -> pipeline202/1(d)
  pipeline153/1(q) -> re_bin_synth0_4/2(data_in)
  pipeline127/1(q) -> pipeline130/1(d)
  pipeline211/1(q) -> re_bin_synth1_4/1(addr)
  pfb_fir1/17(pol2_out8) -> fft1/18(in17)
  Reinterpret25/1 -> pipeline226/1(d)
  comp_add21/1(a+b) -> power22/1
  Delay108/1 -> pipeline146/1(d), bram_mult0_17/1(In1), bram_mult0_24/1(In1), bram_mult0_25/1(In1), bram_mult0_26/1(In1), bram_mult0_27/1(In1), bram_mult0_30/1(In1), bram_mult0_31/1(In1), bram_mult0_18/1(In1), bram_mult0_19/1(In1), bram_mult0_20/1(In1), bram_mult0_21/1(In1), bram_mult0_22/1(In1), bram_mult0_23/1(In1), bram_mult0_29/1(In1), bram_mult0_28/1(In1), bram_mult0_16/1(In1)
  pipeline205/1(q) -> simple_bram_vacc27/1(new_acc)
  Register1/1 -> Relational3/2
  pipeline188/1(q) -> simple_bram_vacc23/1(new_acc)
  Delay68/1 -> round26/1(din)
  pipeline69/1(q) -> comp_add17/1(a)
  fft1/14(out14) -> Delay124/1
  pipeline232/1(q) -> re_bin_synth1_1/3(we)
  round19/1(dout) -> pipeline221/1(d)
  pipeline230/1(q) -> simple_bram_vacc30/2(din)
  Delay67/1 -> round25/1(din)
  pipeline137/1(q) -> pipeline139/1(d)
  comp_add27/1(a+b) -> power29/1
  pipeline180/1(q) -> re_bin_synth0_7/3(we)
  pipeline197/1(q) -> comp_add18/1(a)
  Delay88/1 -> round23/1(din)
  pipeline210/1(q) -> re_bin_synth1_1/2(data_in)
  power17/1 -> Delay114/1
  Reinterpret28/1 -> pipeline140/1(d)
  pipeline215/1(q) -> pipeline217/1(d)
  Reinterpret33/1 -> pipeline184/1(d)
  Delay70/1 -> Delay63/1
  Reinterpret32/1 -> pipeline169/1(d)
  pfb_fir1/15(pol2_out6) -> fft1/16(in15)
  pipeline196/1(q) -> simple_bram_vacc18/1(new_acc)
  pipeline159/1(q) -> re_bin_synth0_3/2(data_in)
  bram_mult0_17/1(c) -> comp_add17/2(b)
  Reinterpret36/1 -> pipeline201/1(d)
  power28/1 -> Delay73/1
  Delay101/1 -> Delay90/1
  bram_mult0_26/1(c) -> comp_add26/2(b)
  acc_cnt1/1(sim_out_reg) -> Terminator48/1
  bram_mult0_23/1(c) -> comp_add23/2(b)
  Delay129/1 -> bram_mult0_19/2(d), pipeline149/1(d)
  simple_bram_vacc24/2(valid) -> pipeline180/1(d)
  fft1/16(out16) -> Delay99/1
  re_bin_synth1_5/1(data_out) -> Terminator41/1
  pipeline142/1(q) -> pipeline147/1(d)
  pipeline173/1(q) -> simple_bram_vacc22/2(din)
  Delay69/1 -> Delay65/1
  Delay74/1 -> Delay79/1
  simple_bram_vacc32/2(valid) -> pipeline85/1(d)
  comp_add16/1(a+b) -> power17/1
  Delay66/1 -> Delay122/1
  round27/1(dout) -> pipeline171/1(d)
  pipeline85/1(q) -> re_bin_synth1_7/3(we)
  pipeline135/1(q) -> re_bin_synth0_0/3(we)
  pipeline206/1(q) -> simple_bram_vacc18/2(din)
  bram_mult0_24/1(c) -> comp_add24/2(b)
  comp_add29/1(a+b) -> power31/1
  pipeline130/1(q) -> simple_bram_vacc31/2(din)
  re_bin_synth1_1/1(data_out) -> Terminator37/1
  Constant8/1 -> fft1/2(shift)
  pipeline155/1(q) -> pipeline158/1(d)
  fft1/8(out06) -> Delay62/1
  bus_expand4/7(out2) -> pfb_fir1/8(pol1_in7)
  Delay76/1 -> acc_cycle1/2
  bus_expand4/3(out6) -> pfb_fir1/4(pol1_in3)
  bram_mult0_25/1(c) -> comp_add25/2(b)
  re_bin_synth0_3/1(data_out) -> Terminator44/1
  comp_add20/1(a+b) -> power21/1
  round23/1(dout) -> pipeline115/1(d)
  pipeline150/1(q) -> re_bin_synth0_1/2(data_in)
  Reinterpret31/1 -> pipeline153/1(d)
  power19/1 -> Delay102/1
  pipeline171/1(q) -> pipeline173/1(d)
  comp_add28/1(a+b) -> power30/1
  fft1/12(out12) -> Delay105/1
  pipeline128/1(q) -> re_bin_synth0_2/1(addr)
  pipeline222/1(q) -> simple_bram_vacc28/1(new_acc)
  bus_expand5/6(out3) -> pfb_fir1/15(pol2_in6)
  comp_add26/1(a+b) -> power28/1
  round29/1(dout) -> pipeline181/1(d)
  pipeline187/1(q) -> pipeline189/1(d)
  Delay42/1 -> bram_mult0_18/2(d), pipeline136/1(d)
  Reinterpret23/1 -> pipeline218/1(d)
  re_bin_synth1_2/1(data_out) -> Terminator38/1
  pipeline136/1(q) -> comp_add24/1(a)
  power30/1 -> Delay82/1
  comp_add23/1(a+b) -> power24/1
  [quant_gain1] -> round16/2(scale), round17/2(scale), round24/2(scale), round25/2(scale), round26/2(scale), round27/2(scale), round28/2(scale), round29/2(scale), round30/2(scale), round31/2(scale), round18/2(scale), round19/2(scale), round20/2(scale), round21/2(scale), round22/2(scale), round23/2(scale)
  Delay71/1 -> Delay67/1
  pipeline145/1(q) -> Delay116/1
  fft1/10(out10) -> Delay103/1
  Delay105/1 -> Delay93/1
  Constant1/1 -> Relational2/2
  bus_expand5/2(out7) -> pfb_fir1/11(pol2_in2)
  Delay78/1 -> round29/1(din)
  pipeline176/1(q) -> comp_add28/1(a)
  Delay109/1 -> Delay108/1
  Delay60/1 -> bram_mult0_22/2(d), pipeline176/1(d)
  Delay92/1 -> Delay78/1
  [sync_gen_1] -> pfb_fir1/1(sync)
  round17/1(dout) -> pipeline186/1(d)
  simple_bram_vacc18/1(dout) -> Reinterpret20/1
  pipeline186/1(q) -> pipeline206/1(d)
  bus_expand5/4(out5) -> pfb_fir1/13(pol2_in4)
  pipeline235/1(q) -> comp_add23/1(a)
  power20/1 -> Delay104/1
  simple_bram_vacc29/1(dout) -> Reinterpret24/1
  bus_expand5/3(out6) -> pfb_fir1/12(pol2_in3)
  pipeline216/1(q) -> simple_bram_vacc29/1(new_acc)
  power24/1 -> Delay115/1
  re_bin_synth1_4/1(data_out) -> Terminator40/1
  fft1/7(out05) -> Delay48/1
  Delay123/1 -> bram_mult0_26/2(d), pipeline212/1(d)
  Delay49/1 -> bram_mult0_23/2(d), pipeline178/1(d)
  spect_cnt1/1 -> pipeline119/1(d), pipeline190/1(d), pipeline193/1(d), pipeline208/1(d), pipeline211/1(d), pipeline224/1(d), pipeline231/1(d), pipeline234/1(d), pipeline192/1(d), pipeline177/1(d), pipeline174/1(d), pipeline167/1(d), pipeline151/1(d), pipeline148/1(d), pipeline128/1(d), pipeline8/1(d)
  Delay86/1 -> acc_cntrl1/2(sync)
  acc_cntrl1/1(new_acc) -> Delay76/1, pipeline132/1(d), pipeline129/1(d), pipeline116/1(d), pipeline229/1(d), pipeline216/1(d), pipeline222/1(d), pipeline205/1(d), pipeline131/1(d), pipeline199/1(d), pipeline182/1(d), pipeline188/1(d), pipeline172/1(d), pipeline156/1(d), pipeline165/1(d), pipeline144/1(d), pipeline196/1(d), pipeline138/1(d)
  simple_bram_vacc30/2(valid) -> pipeline227/1(d)
  Delay124/1 -> Delay123/1
  pipeline138/1(q) -> simple_bram_vacc17/1(new_acc)
  comp_add31/1(a+b) -> power18/1
  Delay110/1 -> Delay98/1
  Delay128/1 -> Delay118/1
  fft1/2(out00) -> Delay70/1
  comp_add22/1(a+b) -> power23/1
  Delay94/1 -> Delay127/1
  bus_expand4/1(msb_out8) -> pfb_fir1/2(pol1_in1)
  simple_bram_vacc26/1(dout) -> Reinterpret35/1
  Delay127/1 -> bram_mult0_27/2(d), pipeline225/1(d)
  simple_bram_vacc28/2(valid) -> pipeline220/1(d)
  re_bin_synth0_2/1(data_out) -> Terminator43/1
  Delay103/1 -> Delay100/1
  pipeline226/1(q) -> re_bin_synth1_5/2(data_in)
  comp_add18/1(a+b) -> power19/1
  [cnt_rst] -> acc_cntr1/1
  munge1/1(dout) -> bus_expand/1(bus_in), bus_expand4/1(bus_in)
  pfb_fir1/11(pol2_out2) -> fft1/12(in11)
  pfb_fir1/12(pol2_out3) -> fft1/13(in12)
  Delay91/1 -> pipeline164/1(d)
  sync_gen1/1(sync_out) -> Delay91/1
  pipeline164/1(q) -> [sync_gen_1]
  ? -> ?
  pipeline237/1(q) -> [acc_len1]
  Constant9/1 -> acc_len_re_bin/1(sim_1)
  acc_len_re_bin/1(in_reg) -> pipeline237/1(d)
  pipeline236/1(q) -> [quant_gain1]
  Constant11/1 -> gain_re_bin/1(sim_1)
  gain_re_bin/1(in_reg) -> pipeline236/1(d)
